simulink model "MCVT_Integration"  (R2020a)
settings: Solver=ode8  StartTime=0.0  StopTime=T_end  FixedStep=dt  RelTol=1e-3  EnableMultiTasking=on
values from neighbouring files (symbols this model uses):
  assumed_power_voltage = 1.25e2    (= 125)    [MCVT_Input_File.m]    (also in MCVT_Run_QuickCheck.m)
  battery_power_toggle = 1    [MCVT_Input_File.m]    (also in MCVT_Run_QuickCheck.m)
  battery_trickle_charge_param = 0.1    [MCVT_Input_File.m]    (also in MCVT_Run_QuickCheck.m)
  battery_trickle_leakage_param = 0.01    [MCVT_Input_File.m]    (also in MCVT_Run_QuickCheck.m)
  damage_case_id = 3    [MCVT_Input_File.m]    (also in MCVT_Run_QuickCheck.m = 1)
  dt = 1/fs    (= 0.001)    [MCVT_Run_QuickCheck.m]    (also in MCVT_Sim_Set.m)
  dt_2 = 1/fs_2    (= 0.001)    [MCVT_Run_QuickCheck.m]    (also in MCVT_Sim_Set.m)
  dt_3 = 1/fs_3    (= 0.001)    [MCVT_Run_QuickCheck.m]    (also in MCVT_Sim_Set.m)
  dt_5 = 1/fs_5    (= 0.005)    [MCVT_Run_QuickCheck.m]    (also in MCVT_Sim_Set.m)
  dt_8 = 1/fs_8    (= 0.05)    [MCVT_Run_QuickCheck.m]    (also in MCVT_Sim_Set.m)
  dt_9 = 1/fs_9    (= 0.001)    [MCVT_Run_QuickCheck.m]    (also in MCVT_Sim_Set.m)
  dust_met_duration = 3    [MCVT_Input_File.m]    (also in MCVT_Run_QuickCheck.m = 2)
  ECLSS_indoor_set_temperature = 298.15    [MCVT_Input_File.m]    (also in MCVT_Run_QuickCheck.m)
  energy_store_max_o = 150    [MCVT_Input_File.m]    (also in MCVT_Run_QuickCheck.m)
  energy_store_o = 120    [MCVT_Input_File.m]    (also in MCVT_Run_QuickCheck.m)
  fs = 1000    [MCVT_Run_QuickCheck.m]    (also in MCVT_Sim_Set.m)
  gain_charge_param = 1    [MCVT_Input_File.m]    (also in MCVT_Run_QuickCheck.m)
  ground_temperature = 373    [MCVT_Habitat_Design_Input_File.m]    (also in MCVT_Run_QuickCheck.m)
  I_max_charge = 800    [MCVT_Input_File.m]    (also in MCVT_Run_QuickCheck.m)
  I_max_discharge = 800    [MCVT_Input_File.m]    (also in MCVT_Run_QuickCheck.m)
  initial_pressure = 1e5    (= 100000)    [MCVT_Input_File.m]    (also in MCVT_Run_QuickCheck.m)
  initial_temperature = 295    [MCVT_Input_File.m]    (also in MCVT_Run_QuickCheck.m)
  interior_leak_rate = 0.00415    [MCVT_Input_File.m]    (also in MCVT_Run_QuickCheck.m)
  launch_dust_accum_start_time = 10    [MCVT_Input_File.m]    (also in MCVT_Run_QuickCheck.m = 2)
  launch_dust_accum_stop_time = 12    [MCVT_Input_File.m]    (also in MCVT_Run_QuickCheck.m = 3)
  meteorite_impact_time = 2    [MCVT_Input_File.m]    (also in MCVT_Run_QuickCheck.m = 1)
  nuclear_power_toggle = 1    [MCVT_Input_File.m]    (also in MCVT_Run_QuickCheck.m)
  print_interval = 30    [MCVT_Input_File.m]
  SOC_lower_thres = 0.5    [MCVT_Input_File.m]    (also in MCVT_Run_QuickCheck.m)
  SOC_upper_thres = 0.9    [MCVT_Input_File.m]    (also in MCVT_Run_QuickCheck.m)
  solar_power_toggle = 1    [MCVT_Input_File.m]    (also in MCVT_Run_QuickCheck.m)
  space_temperature = 4    [MCVT_Habitat_Design_Input_File.m]    (also in MCVT_Run_QuickCheck.m)
  Start_angle = 90    [MCVT_Input_File.m]    (also in MCVT_Run_QuickCheck.m)
undefined symbols (not defined in the file or in its folder): A_flow_restrictor, ACTIVITY_LOOKUP_TABLE, air, Aref, Bmap, Bmap2, Bmap3, comms, DiscIntNeverNeededParam, DmgSPL, dust_parameters, ECLSS_dust_damage_repair_time, ECLSS_heater_capacity, ECLSS_IPS_restrictor, ECLSS_IPS_setpointPressure, ECLSS_paint_damage_repair_time, ECLSS_radiatorLoop_discreteNumber, ECLSS_Vst, emptyActivity, expected_heat_add, FI, habitat, HIEM2SMM, HIEM2STM, HIEMDamageElements, impact_numbering, initial_SPLT_damage_index, input_air, input_Cond, input_CP, input_discretization, input_EV, input_Evap, input_radiatorLoop, K_ECLSS_dust_single, K_ECLSS_paint_single, K_Structure_damage_single, Kref, kW_to_W, meteoroidDustDep, mFRF, ndelays, nominalDustDep, noverlap, nuclear_toggle_gain, output_limits, panel_paint0, Ps0, reference_temperature, RepairTime_ECLSS_factor, Sensor_Location_SMM, SMM_T0, SMM_T1, SMM_T2, SMM_T3, SMM_T4, SMM_T4_0, SMM_T4_1, SMM_T4_2, solar_FDD_K ... (+73)
libraries: neural x350, simulink x39, xpcutilitieslib x7, xpcrtudplib x7, dspstat3 x1
(part 1 of 43)
settings Model: Creator=cjpan  LastModifiedBy=Awareness
settings SolverCC: ConcurrentTasks=on  AutoInsertRateTranBlk=on
settings DataIOCC: SaveFormat=StructureWithTime  ReturnWorkspaceOutputs=off
settings OptimizationCC: BlockReduction=off  OptimizeBlockIOStorage=off  BufferReuse=off  GlobalBufferReuse=off  ExpressionFolding=off  LocalBlockOutputs=off  OptimizeDataStoreBuffers=off  BusAssignmentInplaceUpdate=off
settings DebuggingCC: ArithmeticOperatorsInVariantConditions=warning
settings SFSimCC: CustomCodeUndefinedFunction=UseInterfaceOnly
settings RTWCC: Description=Generic Real-Time Target  RTWVerbose=off  BuildConfiguration=Faster Runs  RTWCompilerOptimization=on
settings GRTTargetCC: TargetLangStandard=C89/C90 (ANSI)

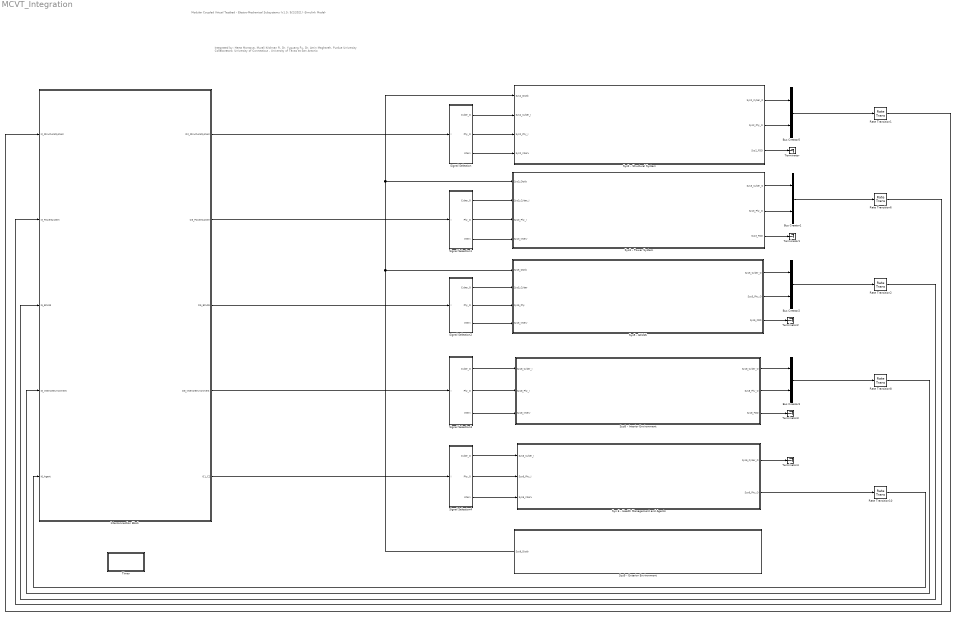
== system MCVT_Integration ==
  note: Modular Coupled Virtual Testbed - Electro-Mechanical Subsystems (v1.5: 9/2/2021)
      | -Simulink Model-
  note: Integrated by: Herta Montoya, Murali Krishnan R, Dr. Yuguang Fu, Dr. Amin Maghareh, Purdue University
      | Collaborators: University of Connecticut , University of Texas at San Antonio
  Bus Creator{1..3,5} (4 alike) : BusCreator  Inputs=2
  Interconnection Block : Subsystem  mask(Interconnection Block){}
  Rate Transition{1..2,6,8,10} (5 alike) : RateTransition  OutPortSampleTime=dt
  Signal Selection : Subsystem
  Signal Selection1 : Subsystem
  Signal Selection2 : Subsystem
  Signal Selection3 : Subsystem
  Signal Selection4 : Subsystem
  Sys 6 - Health Management and Agents : Subsystem  mask(Agents and C2){}
      mask help: Provide a summary of functionalities here
  Sys2 - Structural System : Subsystem  mask(Subsystem 2: Structure){}
      mask help:
      | - Structural Mechanical
      | - Structural Thermal
      | - Structural Protective Layer
      | - Structural Mechanical FDD
  Sys3 - Power System : Subsystem  mask(Subsystem 3: Power){}
      mask help: Provide a summary of functionalities here
  Sys5 - ECLSS : Subsystem  mask(Subsystem 5: ECLSS){}
      mask help: Provide a summary of functionalities here
  Sys8 - Interior Environment : Subsystem  mask(Subsystem 8: Interior Environment){}
      mask help: Provide a summary of functionalities here
  Sys9 - Exterior Environment : Subsystem  mask(Subsystem 9: Exterior Environment){}
      mask help: Provide a summary of functionalities here
  Terminator{,1..4} (5 alike) : Terminator
  Timer : Subsystem
  wires:
  Rate Transition2/1 -> Interconnection Block/3(I5_ECLSS)
  Rate Transition6/1 -> Interconnection Block/2(I3_PowerSystem)
  Rate Transition8/1 -> Interconnection Block/4(I8_InteriorEnvironment)
  Rate Transition10/1 -> Interconnection Block/5(I6_Agent)
  Sys9 - Exterior Environment/1(Sys9_Distb) -> Sys2 - Structural System/1(Sys2_Distb), Sys3 - Power System/1(Sys3_Distb), Sys5 - ECLSS/1(Sys5_Distb)
  Sys 6 - Health Management and Agents/2(Sys6_Phy_O) -> Rate Transition10/1
  Interconnection Block/2(O3_PowerSystem) -> Signal Selection1/1(I)
  Bus Creator5/1 -> Rate Transition1/1
  Sys2 - Structural System/2(Sys2_Phy_O) -> Bus Creator5/2
  Sys2 - Structural System/1(Sys2_Cyber_O) -> Bus Creator5/1
  Sys3 - Power System/1(Sys3_Cyber_O) -> Bus Creator1/1
  Sys3 - Power System/2(Sys3_Phy_O) -> Bus Creator1/2
  Bus Creator1/1 -> Rate Transition6/1
  Sys5 - ECLSS/2(Sys5_Phy_O) -> Bus Creator2/2
  Sys5 - ECLSS/1(Sys5_Cyber_O) -> Bus Creator2/1
  Bus Creator2/1 -> Rate Transition2/1
  Signal Selection/2(Phy_O) -> Sys2 - Structural System/3(Sys2_Phy_I)
  Signal Selection/3(Interv) -> Sys2 - Structural System/4(Sys2_Interv)
  Signal Selection/1(Cyber_O) -> Sys2 - Structural System/2(Sys2_Cyber_I)
  Signal Selection1/1(Cyber_O) -> Sys3 - Power System/2(Sys3_Cyber_I)
  Signal Selection1/2(Phy_O) -> Sys3 - Power System/3(Sys3_Phy_I)
  Signal Selection1/3(Interv) -> Sys3 - Power System/4(Sys3_Interv)
  Interconnection Block/3(O5_ECLSS) -> Signal Selection2/1(I)
  Signal Selection2/1(Cyber_O) -> Sys5 - ECLSS/2(Sys5_Cyber)
  Signal Selection2/2(Phy_O) -> Sys5 - ECLSS/3(Sys5_Phy)
  Interconnection Block/4(O8_InteriorEnvrionment) -> Signal Selection3/1(I)
  Signal Selection3/1(Cyber_O) -> Sys8 - Interior Environment/1(Sys8_Cyber_I)
  Signal Selection3/2(Phy_O) -> Sys8 - Interior Environment/2(Sys8_Phy_I)
  Signal Selection3/3(Interv) -> Sys8 - Interior Environment/3(Sys8_Interv)
  Interconnection Block/5(O1_C2) -> Signal Selection4/1(I)
  Sys8 - Interior Environment/1(Sys8_Cyber_O) -> Bus Creator3/1
  Sys8 - Interior Environment/2(Sys8_Phy_O) -> Bus Creator3/2
  Bus Creator3/1 -> Rate Transition8/1
  Signal Selection4/1(Cyber_O) -> Sys 6 - Health Management and Agents/1(Sys6_Cyber_I)
  Signal Selection4/2(Phy_O) -> Sys 6 - Health Management and Agents/2(Sys6_Phy_I)
  Signal Selection4/3(Interv) -> Sys 6 - Health Management and Agents/3(Sys6_Interv)
  Rate Transition1/1 -> Interconnection Block/1(I2_StructuralSystem)
  Interconnection Block/1(O2_StructuralSystem) -> Signal Selection/1(I)
  Sys2 - Structural System/3(Sys2_FDD) -> Terminator/1
  Sys3 - Power System/3(Sys3_FDD) -> Terminator1/1
  Sys5 - ECLSS/3(Sys5_FDD) -> Terminator2/1
  Sys8 - Interior Environment/3(Sys8_FDD) -> Terminator3/1
  Sys 6 - Health Management and Agents/1(Sys6_Cyber_O) -> Terminator4/1
  Signal Selection2/3(Interv) -> Sys5 - ECLSS/4(Sys5_Interv)

== system MCVT_Integration/Interconnection Block ==
  note: - System Interdependencies | Signal Exchange -
  note: Outputs from the Interconnection Block to the Structural System
  note: Interconnection Block
  note: Inputs from the Structural System to the Interconnection Block
  note: Inputs from the Power System to the Interconnection Block
  note: Outputs from the Interconnection Block to the Power System
  note: Inputs from ECLSS to the Interconnection Block
  note: Outputs from the Interconnection Block to ECLSS
  note: Inputs from the Interior Environment to the Interconnection Block
  note: Outputs from the Interconnection Block to the Interior Environment
  note: Outputs from the Interconnection Block to C2/Agent
  note: Inputs from the Agent to the Interconnection Block
  I2_StructuralSystem : Inport
  I3_PowerSystem : Inport  Port=2
  I5_ECLSS : Inport  Port=3
  I8_InteriorEnvironment : Inport  Port=4
  I6_Agent : Inport  Port=5
  Bus Creator : BusCreator  Inputs=4
  Bus Creator1 : BusCreator  Inputs=5
  Bus Creator2 : BusCreator  Inputs=10
  Bus Creator3 : BusCreator  Inputs=5
  Bus Selector : BusSelector  OutputSignals=Sys3_PhyO.Sys3_Out_Power_Supply_to_Sys2_kW_
  Bus Selector1 : BusSelector  OutputSignals=Sys8_PhyO.Sys8_Out_TempWall,Sys8_PhyO.Sys8_Out_PressureWall
  Bus Selector10 : BusSelector  OutputSignals=Sys8_CyberO.Sys8_Out_PSTemp,Sys8_PhyO.Sys8_Out_Airflow,Sys8_CyberO.Sys8_Out_PCPressure
  Bus Selector11 : BusSelector  OutputSignals=AgentComms.Repair_Comms_NPG,AgentComms.Repair_Comms_SPG
  Bus Selector12 : BusSelector  OutputSignals=Sys2_CyberO.Sys2_out_PowerConsumption
  Bus Selector2 : BusSelector  OutputSignals=Sys3_PhyO.Sys3_Out_Power_Supply_to_Sys5_kW_
  Bus Selector3 : BusSelector  OutputSignals=AgentComms.Repair_Comms_Structure
  Bus Selector4 : BusSelector  OutputSignals=Sys3_PhyO.Sys3_Out_Power_Supply_to_Sys8_kW_
  Bus Selector5 : BusSelector  OutputSignals=Sys5_PhyO.Sys5_Out_Airflow,Sys5_PhyO.Sys5_Out_Temp,Sys5_PhyO.Sys5_Air_Vent,Sys5_PhyO.Sys5_Air_Supply,Sys5_PhyO.Sys5_Out_HeatingEnergy,Sys5_PhyO.Sys5_Out_Heat
  Bus Selector6 : BusSelector  OutputSignals=AgentComms.Repair_Comms_ECLSS
  Bus Selector7 : BusSelector  OutputSignals=Sys2_PhyO.Sys2_out_InteriorWallSurfTemp,Sys2_PhyO.Sys2_out_Damage,Sys2_PhyO.Sys2_out_ExteriorWallSurfTemp
  Bus Selector8 : BusSelector  OutputSignals=Sys5_CyberO.Sys5_Out_PowerRequest
  Bus Selector9 : BusSelector  OutputSignals=Sys8_CyberO.Sys8_Out_PowerConsumption
  C2 Connection Handler : Subsystem
  O2_StructuralSystem : Outport
  O3_PowerSystem : Outport  Port=2
  O5_ECLSS : Outport  Port=3
  O8_InteriorEnvrionment : Outport  Port=4
  O1_C2 : Outport  Port=5
  wires:
  I3_PowerSystem/1 -> [Power]
  I8_InteriorEnvironment/1 -> [InteriorEnv]
  Bus Selector/1 -> Bus Creator/1  "<Sys3_Out_Power_Supply_to_Sys2_kW_>"
  Bus Selector1/1 -> Bus Creator/2  "<Sys8_Out_TempWall>"
  Bus Creator/1 -> O2_StructuralSystem/1
  Bus Selector2/1 -> Bus Creator1/1  "<Sys3_Out_Power_Supply_to_Sys5_kW_>"
  Bus Creator1/1 -> O5_ECLSS/1
  I5_ECLSS/1 -> [ECLSS]
  Bus Creator2/1 -> O8_InteriorEnvrionment/1
  I2_StructuralSystem/1 -> [Structural]
  Bus Selector4/1 -> Bus Creator2/4  "<Sys3_Out_Power_Supply_to_Sys8_kW_>"
  Bus Selector5/1 -> Bus Creator2/5  "<Sys5_Out_Airflow>"
  Bus Selector5/2 -> Bus Creator2/6  "<Sys5_Out_Temp>"
  Bus Selector8/1 -> Bus Creator3/2  "<Sys5_Out_PowerRequest>"
  Bus Selector9/1 -> Bus Creator3/3  "<Sys8_Out_PowerConsumption>"
  Bus Creator3/1 -> O3_PowerSystem/1
  Bus Selector5/3 -> Bus Creator2/7  "<Sys5_Air_Vent>"
  Bus Selector5/4 -> Bus Creator2/8  "<Sys5_Air_Supply>"
  Bus Selector10/1 -> Bus Creator1/2  "<Sys8_Out_PSTemp>"
  Bus Selector10/2 -> Bus Creator1/3  "<Sys8_Out_Airflow>"
  Bus Selector10/3 -> Bus Creator1/4  "<Sys8_Out_PCPressure>"
  Bus Selector5/5 -> Bus Creator2/9  "<Sys5_Out_HeatingEnergy>"
  Bus Selector1/2 -> Bus Creator/3  "<Sys8_Out_PressureWall>"
  I6_Agent/1 -> [Agent]
  Bus Selector3/1 -> Bus Creator/4  "<Repair_Comms_Structure>"
  Bus Selector11/1 -> Bus Creator3/4  "<Repair_Comms_NPG>"
  Bus Selector11/2 -> Bus Creator3/5  "<Repair_Comms_SPG>"
  C2 Connection Handler/1(O1_C2) -> O1_C2/1
  Bus Selector5/6 -> Bus Creator2/10  "<Sys5_Out_Heat>"
  [Structural] -> Bus Selector12/1
  Bus Selector12/1 -> Bus Creator3/1  "<Sys2_out_PowerConsumption>"
  [Structural] -> Bus Selector7/1
  Bus Selector7/1 -> Bus Creator2/1  "<Sys2_out_InteriorWallSurfTemp>"
  Bus Selector7/2 -> Bus Creator2/2  "<Sys2_out_Damage>"
  Bus Selector7/3 -> Bus Creator2/3  "<Sys2_out_ExteriorWallSurfTemp>"
  [Structural] -> C2 Connection Handler/1(I2_StructuralSystem)
  [Power] -> Bus Selector/1
  [Power] -> Bus Selector2/1
  [Power] -> Bus Selector4/1
  [Power] -> C2 Connection Handler/2(I2_PowerSystem)
  [ECLSS] -> Bus Selector8/1
  [ECLSS] -> Bus Selector5/1
  [ECLSS] -> C2 Connection Handler/3(I5_ECLSS)
  [InteriorEnv] -> Bus Selector1/1
  [InteriorEnv] -> Bus Selector9/1
  [InteriorEnv] -> Bus Selector10/1
  [Agent] -> Bus Selector3/1
  [Agent] -> Bus Selector11/1
  [Agent] -> Bus Selector6/1
  Bus Selector6/1 -> Bus Creator1/5  "<Repair_Comms_ECLSS>"

== system MCVT_Integration/Signal Selection ==
  I : Inport
  Bus Creator : BusCreator  Inputs=3
  Bus Selector : BusSelector  OutputSignals=Sys8_Out_TempWall,Sys8_Out_PressureWall,Sys3_Out_Power_Supply_to_Sys2_kW_
  Bus Selector1 : BusSelector  OutputSignals=Repair_Comms_Structure
  Cyber_O : Outport
  Phy_O : Outport  Port=2
  Interv : Outport  Port=3
  wires:
  Bus Selector1/1 -> Interv/1  "<Repair_Comms_Structure>"
  I/1 -> Bus Selector1/1, Bus Selector/1
  Bus Creator/1 -> Phy_O/1
  Bus Selector/1 -> Bus Creator/1  "<Sys8_Out_TempWall>"
  Bus Selector/2 -> Bus Creator/2  "<Sys8_Out_PressureWall>"
  Bus Selector/3 -> Bus Creator/3  "<Sys3_Out_Power_Supply_to_Sys2_kW_>"

== system MCVT_Integration/Signal Selection1 ==
  I : Inport
  Bus Creator : BusCreator  Inputs=3
  Bus Creator1 : BusCreator  Inputs=2
  Bus Selector : BusSelector  OutputSignals=Sys2_out_PowerConsumption,Sys5_Out_PowerRequest,Sys8_Out_PowerConsumption
  Bus Selector1 : BusSelector  OutputSignals=Repair_Comms_NPG,Repair_Comms_SPG
  Cyber_O : Outport
  Phy_O : Outport  Port=2
  Interv : Outport  Port=3
  wires:
  I/1 -> Bus Selector/1, Bus Selector1/1
  Bus Selector/1 -> Bus Creator/1  "<Sys2_out_PowerConsumption>"
  Bus Selector/2 -> Bus Creator/2  "<Sys5_Out_PowerRequest>"
  Bus Selector/3 -> Bus Creator/3  "<Sys8_Out_PowerConsumption>"
  Bus Creator/1 -> Cyber_O/1
  Bus Selector1/1 -> Bus Creator1/1  "<Repair_Comms_NPG>"
  Bus Selector1/2 -> Bus Creator1/2  "<Repair_Comms_SPG>"
  Bus Creator1/1 -> Interv/1

== system MCVT_Integration/Signal Selection2 ==
  I : Inport
  Bus Creator : BusCreator  Inputs=2
  Bus Creator1 : BusCreator  Inputs=2
  Bus Creator2 : BusCreator  Inputs=2
  Bus Selector : BusSelector  OutputSignals=Sys8_Out_PSTemp,Sys8_Out_PCPressure
  Bus Selector1 : BusSelector  OutputSignals=Sys3_Out_Power_Supply_to_Sys5_kW_,Sys8_Out_Airflow
  Bus Selector2 : BusSelector  OutputSignals=Repair_Comms_ECLSS
  Subsystem : Subsystem
  Cyber_O : Outport
  Phy_O : Outport  Port=2
  Interv : Outport  Port=3
  wires:
  I/1 -> Bus Selector1/1, Bus Selector/1, Bus Selector2/1
  Bus Selector/1 -> Bus Creator/1  "<Sys8_Out_PSTemp>"
  Bus Selector/2 -> Bus Creator/2  "<Sys8_Out_PCPressure>"
  Bus Creator/1 -> Cyber_O/1
  Bus Selector1/1 -> Bus Creator1/1  "<Sys3_Out_Power_Supply_to_Sys5_kW_>"
  Bus Selector1/2 -> Bus Creator1/2  "<Sys8_Out_Airflow>"
  Bus Creator1/1 -> Phy_O/1
  Bus Creator2/1 -> Interv/1  "Sys5_Interv"
  Bus Selector2/1 -> Subsystem/1(<Repair_Comms_ECLSS>)  "<Repair_Comms_ECLSS>"
  Subsystem/1(Repair_ECLSS_Dust) -> Bus Creator2/1
  Subsystem/2(Repair_ECLSS_Paint) -> Bus Creator2/2

== system MCVT_Integration/Signal Selection3 ==
  I : Inport
  Cyber_O : Outport
  Phy_O : Outport  Port=2
  Interv : Outport  Port=3
  wires:
  I/1 -> Phy_O/1

== system MCVT_Integration/Signal Selection4 ==
  I : Inport
  Bus Selector : BusSelector  OutputSignals=Sys6_Cyber,Sys6_Physical
  Cyber_O : Outport
  Phy_O : Outport  Port=2
  Interv : Outport  Port=3
  wires:
  I/1 -> Bus Selector/1
  Bus Selector/1 -> Cyber_O/1  "<Sys6_Cyber>"
  Bus Selector/2 -> Phy_O/1  "<Sys6_Physical>"

== system MCVT_Integration/Sys 6 - Health Management and Agents ==
  note: Coded by: Murali Krishnan Rajasekharan Pillai
      | Affiliations: Purdue University
      | Funded by: NASA STRI - RETH Institute
      |
      | Current Features (for the Agent Model):
      | - Single Agent Model with Finite Memory and FIFO Scheduling with no over-ride
      | - Agent States : [soc, position, mobility-HS]
      | - Agent Interactions : [Nuclear PG, Solar PG, Structure, ECLSS, Inventory]
      |
      | Current featurers (for the Decision Maker)
      | - FIFO scheduler which cann potentially re-schedule activities if FDD component doesn't react fast to the intervention
      | - No over-ride functionality covered (aka, cannot `cancel` an intervention), except at Habitat design level (aka, within the `MCVT_Habitat_Input_File`)
  note: Health Management System and Agent Model
  note: Systems Outputs to File
  Sys6_Cyber_I : Inport
  Sys6_Phy_I : Inport  Port=2
  Sys6_Interv : Inport  Port=3
  " Agent System" : Subsystem
  Bus Creator : BusCreator  Inputs=1
  Bus Creator1 : BusCreator  Inputs=1
  Bus Creator2 : BusCreator  Inputs=2
  Decision Maker : Subsystem
      settings: PortSchema="{\"entries\":[{\"content\":{\"sides\":[{\"content\":{\"connectorIds\":[\"In1\"]},\"type\":\"ConnectorPlacement.EquallySpacedRectSide\",\"uuid\":\"af73862b-44c1-41ec-b3ca-270812b76194\"},{\"content\":{\"connectorIds\":[\"In2\",\"In3\"],\"side\":\"RIGHT\"},\"type\":\"ConnectorPlacement.EquallySpacedRectSide\",\"uuid\":\"9454815f-a3fd-4c8a-9f4b-2b34d3ab7b42\"},{\"content\":{\"connectorIds\":[],\"side\":\"TOP\"},\"type\":\"ConnectorPlacement.EquallySpacedRectSide\",\"uuid\":\"90a25189-81d9-48d5-b6fa-d5f2bdc0713f\"},{\"content\":{\"connectorIds\":[\"Out1\"],\"side\":\"BOTTOM\"},\"type\":\"ConnectorPlacement.EquallySpacedRectSide\",\"uuid\":\"1751f83f-c6df-4ec8-9c75-9827cc634605\"}]},\"type\":\"ConnectorPlacement.EquallySpacedConnector\",\"uuid\":\"55de7abc-ed98-425f-94f2-679ed52f4cec\"}],\"packageUris\":[\"http://schema.mathworks.com/mf0/Connector/19700101\"],\"version\":\"1.0\"}"
  Rate Transition1 : RateTransition  OutPortSampleTime=dt_6
  Rate Transition9 : RateTransition  OutPortSampleTime=dt_6
  Terminator2 : Terminator
  To File : ToFile  Filename=Sys6_out.mat  MatrixName=sys6_out  SaveFormat=Timeseries
  Sys6_Cyber_O : Outport
  Sys6_Phy_O : Outport  Port=2
  wires:
  Rate Transition9/1 -> Decision Maker/3(Sub6_Cyber_Inputs)
  " Agent System"/1(AgentStates) -> Decision Maker/1(Agent_States), Bus Creator/1  "Agent_States"
  Sys6_Cyber_I/1 -> Rate Transition9/1
  Sys6_Interv/1 -> Terminator2/1
  Bus Creator/1 -> Sys6_Cyber_O/1, [Sys6_CyberO]  "Sys6_CyberO"
  Decision Maker/1(Intervention_Pgm) -> " Agent System"/1(Intervention_Pgm)
  Sys6_Phy_I/1 -> Rate Transition1/1
  Bus Creator1/1 -> [Sys6_PhysO], Sys6_Phy_O/1  "Sys6_Phy_O"
  " Agent System"/2(AgentComms) -> Bus Creator1/1  "AgentComms"
  Bus Creator2/1 -> To File/1
  [Sys6_CyberO] -> Bus Creator2/1  "Sys6_CyberO"
  [Sys6_PhysO] -> Bus Creator2/2  "Sys6_PhyO"
  Rate Transition1/1 -> Decision Maker/2(Sys6_Phy_Inputs)

== system MCVT_Integration/Sys2 - Structural System ==
  note: Coded by: Dr . Seungwook Seok, Adnan Shahriar, Sterling Reynolds, Sachin Tripathi, Jeffrey Steiner, Zixin Wang
      | Affiliations: University of Texas at San Antonio , University of Connecticut, Purdue University
  note: Structural System
      | - Simulink Model -
  note: Systems Outputs to File
  Sys2_Distb : Inport
  Sys2_Cyber_I : Inport  Port=2
  Sys2_Phy_I : Inport  Port=3
  Sys2_Interv : Inport  Port=4
  Bus Creator1 : BusCreator  Inputs=3
  Bus Creator2 : BusCreator  Inputs=2
  Bus Creator6 : BusCreator  Inputs=4
  Bus Selector : BusSelector  OutputSignals=SolarFluxSpatial,GroundAcc_xyz,MetCord_xyz,FI_index,dmg_caseo
  Bus Selector1 : BusSelector  OutputSignals=Sys8_Out_TempWall,Sys8_Out_PressureWall,Sys3_Out_Power_Supply_to_Sys2_kW_
  Rate Transition1 : RateTransition  OutPortSampleTime=dt_2
  Rate Transition2 : RateTransition  OutPortSampleTime=dt_2
  Rate Transition3 : RateTransition  OutPortSampleTime=dt_2
  Sys2_Structural_System : Subsystem
  Terminator : Terminator
  To File : ToFile  Filename=Sys2_out.mat  MatrixName=sys2_out  SaveFormat=Timeseries
  power2 : MATLAB Function  TreatAsAtomicUnit=on
  Sys2_Cyber_O : Outport
  Sys2_Phy_O : Outport  Port=2
  Sys2_FDD : Outport  Port=3
  wires:
  Sys2_Structural_System/7(Sys2_out_Total_Damage [-]) -> Bus Creator6/4  "Sys2_Total_Damage_to_Sys6"
  Rate Transition3/1 -> Sys2_Structural_System/9(Sys2_In_Repair_Status [-])
  Rate Transition1/1 -> Bus Selector/1
  Bus Creator1/1 -> [Sys2_CyberO], Sys2_Cyber_O/1  "Sys2_CyberO"
  Bus Selector/4 -> Sys2_Structural_System/4(Sys2_In_FI_Index [-])  "<FI_index>"
  Bus Selector1/2 -> Sys2_Structural_System/7(Sys2_In_Internal_Pressure [Pa])  "<Sys8_Out_PressureWall>"
  Rate Transition2/1 -> Bus Selector1/1
  Bus Selector/3 -> Sys2_Structural_System/3(Sys2_In_Meteoroid_Location [m])  "<MetCord_xyz>"
  Bus Selector1/1 -> Sys2_Structural_System/6(Sys2_In_Interior_Surface_Temperature [K])  "<Sys8_Out_TempWall>"
  Sys2_Structural_System/4(Sys2_out_InteriorWallSurfaceTemp [K]) -> Bus Creator6/1  "Sys2_out_InteriorWallSurfTemp"
  Sys2_Structural_System/5(Sys2_out_Damage [-]) -> Bus Creator6/2  "Sys2_out_Damage"
  Sys2_Distb/1 -> Rate Transition1/1
  Bus Selector/1 -> Sys2_Structural_System/1(Sys2_In_Solar_Irradiation [W/m^2])  "<SolarFluxSpatial>"
  Bus Selector/5 -> Sys2_Structural_System/5(Sys2_In_dmg_case [-])  "<dmg_caseo>"
  Sys2_Structural_System/2(Sys2_Out_Damage_Information [-]) -> [Sys2_FDD_Info], Bus Creator1/2  "Sys2_out_DamageProbabilities"
  power2/1(Y) -> Bus Creator1/3  "Sys2_out_PowerConsumption"
  Sys2_Phy_I/1 -> Rate Transition2/1
  Sys2_Structural_System/6(Sys2_out_ExteriorWallTemp [K]) -> Bus Creator6/3  "Sys2_out_ExteriorWallSurfTemp"
  Sys2_Structural_System/1(Sys2_out_InteriorWallSurfaceTemp_Sensors [K]) -> Bus Creator1/1  "Sys2_out_InteriorWallSurfTemp_ Sensors"
  Sys2_Structural_System/3(Sys2_out_PowerConsumption [kW]) -> power2/1(x)
  Sys2_Cyber_I/1 -> Terminator/1
  Sys2_Interv/1 -> Rate Transition3/1
  Bus Creator6/1 -> [Sys2_PhysO], Sys2_Phy_O/1  "Sys2_PhyO"
  Bus Selector1/3 -> Sys2_Structural_System/8(Sys2_In_Power_Supply [kW])  "<Sys3_Out_Power_Supply_to_Sys2_kW_>"
  Bus Selector/2 -> Sys2_Structural_System/2(Sys2_In_Moonquake_Acceleration [m/s^2])  "<GroundAcc_xyz>"
  Bus Creator2/1 -> To File/1
  [Sys2_FDD_Info] -> Sys2_FDD/1  "Sys2_FDD"
  [Sys2_PhysO] -> Bus Creator2/2  "Sys2_PhyO"
  [Sys2_CyberO] -> Bus Creator2/1  "Sys2_CyberO"

== system MCVT_Integration/Sys3 - Power System ==
  note: Systems Outputs to File
  note: Coded by: Ryan Tomastik, Kairui Hao
      | Affiliations: University of Connecticut, Purdue University
  note: Power System
      | -Simulink Model-
  Sys3_Distb : Inport
  Sys3_Cyber_I : Inport  Port=2
  Sys3_Phy_I : Inport  Port=3
  Sys3_Interv : Inport  Port=4
  Bus Creator2 : BusCreator  Inputs=2
  Bus Creator3 : BusCreator  Inputs=5
  Bus Creator4 : BusCreator  Inputs=2
  Bus Selector1 : BusSelector  OutputSignals=DustAccu,SolarFlux
  Bus Selector6 : BusSelector  OutputSignals=Sys2_out_PowerConsumption,Sys5_Out_PowerRequest,Sys8_Out_PowerConsumption
  Demux : Demux  Outputs=3
  Discrete-Time Integrator : DiscreteIntegrator  SampleTime=-1  ICPrevOutput=DiscIntNeverNeededParam  ICPrevScaledInput=DiscIntNeverNeededParam
  Power Generation Subsystem1 : Subsystem
  Rate Transition1 : RateTransition  OutPortSampleTime=dt_3
  Rate Transition4 : RateTransition  OutPortSampleTime=dt_3
  Rate Transition5 : RateTransition  OutPortSampleTime=dt_3
  Terminator : Terminator
  Terminator3 : Terminator
  To File : ToFile  Filename=Sys3_out.mat  MatrixName=sys3_out  SaveFormat=Timeseries
  Sys3_Cyber_O : Outport
  Sys3_Phy_O : Outport  Port=2
  Sys3_FDD : Outport  Port=3
  wires:
  Power Generation Subsystem1/3(Sys3_Out_Power_Supply_to_Sys8 [kW]) -> Bus Creator3/3  "Sys3_Out_Power_Supply_to_Sys8_kW_"
  Power Generation Subsystem1/2(Sys3_Out_Power_Supply_to_Sys5 [kW]) -> Bus Creator3/2  "Sys3_Out_Power_Supply_to_Sys5_kW_"
  Sys3_Cyber_I/1 -> Rate Transition5/1
  Demux/3 -> Terminator/1
  Sys3_Phy_I/1 -> Terminator3/1
  Power Generation Subsystem1/7(Sys3_Out_Current_Power_Stored [kWh]) -> Bus Creator2/2  "Sys3_Out_PowerStorage"
  Bus Creator2/1 -> [Sys3_CyberO], Sys3_Cyber_O/1  "Sys3_CyberO"
  Bus Selector6/2 -> Power Generation Subsystem1/5(Sys3_In_Power_Consumed_by_Sys5 [kW])  "<Sys5_Out_PowerRequest>"
  Bus Selector1/2 -> Power Generation Subsystem1/3(Sys3_In_Solar_Flux [W/m^2])  "<SolarFlux>"
  Bus Selector6/3 -> Power Generation Subsystem1/6(Sys3_In_Power_Consumed_by_Sys8 [kW])  "<Sys8_Out_PowerConsumption>"
  Rate Transition1/1 -> Power Generation Subsystem1/7(Sys3_In_Intervention)
  Bus Selector1/1 -> Demux/1  "<DustAccu>"
  [Sys3_CyberO] -> Bus Creator4/1  "Sys3_CyberO"
  Bus Creator4/1 -> To File/1
  Demux/2 -> Power Generation Subsystem1/2(Sys3_In_Dust_Accumulation_PV [mg/cm^2])
  Bus Selector6/1 -> Power Generation Subsystem1/4(Sys3_In_Power_Consumed_by_Sys2 [kW])  "<Sys2_out_PowerConsumption>"
  Power Generation Subsystem1/4(Sys3_Out_total_power [kW]) -> Bus Creator3/4  "Sys3_Out_Total_Power_Generated_kW_"
  Rate Transition4/1 -> Bus Selector1/1
  Sys3_Interv/1 -> Rate Transition1/1
  Power Generation Subsystem1/6(Sys3_Out_Damage_Information) -> [Sys3_FDD_Info], Bus Creator2/1  "Sys3_Out_PowerSystemDamageInformation"
  Sys3_Distb/1 -> Rate Transition4/1
  Bus Creator3/1 -> Sys3_Phy_O/1, [Sys3_PhysO]  "Sys3_PhyO"
  Power Generation Subsystem1/1(Sys3_Out_Power_Supply_to_Sys2 [kW]) -> Bus Creator3/1  "Sys3_Out_Power_Supply_to_Sys2_kW_"
  Power Generation Subsystem1/5(Sys3_Out_Physical_Inputs_Agents) -> Bus Creator3/5  "Sys3_Out_Physical_Inputs_Agents"
  Demux/1 -> Discrete-Time Integrator/1
  [Sys3_PhysO] -> Bus Creator4/2  "Sys3_PhyO"
  Rate Transition5/1 -> Bus Selector6/1
  Discrete-Time Integrator/1 -> Power Generation Subsystem1/1(Sys3_In_Dust_Accumulation_Nuclear [mg/cm^2])
  [Sys3_FDD_Info] -> Sys3_FDD/1  "Sys3_FDD"

== system MCVT_Integration/Sys5 - ECLSS ==
  note: Environmental Control and Life Support System
      | - Simulink Model -
  note: Coded by: Jaewon Park, Dr. C.J. Pan, Drake Hart
      | Affiliations: P urdue University
  note: %%%%todo%%%%
  note: Systems Outputs to File
  Sys5_Distb : Inport
  Sys5_Cyber : Inport  Port=2
  Sys5_Phy : Inport  Port=3
  Sys5_Interv : Inport  Port=4
  Active Thermal Control System (ATCS) : Subsystem
  Add : Sum
  Allocate Power : Sum  Inputs=+-
  Bus Creator1 : BusCreator  Inputs=2
  Bus Creator8 : BusCreator  Inputs=3
  Bus Creator9 : BusCreator  Inputs=6
  Bus Selector1 : BusSelector  OutputSignals=Sys8_Out_PSTemp,Sys8_Out_PCPressure
  Bus Selector2 : BusSelector  OutputSignals=SolarFlux,DustAccu,dmg_caseo
  Bus Selector3 : BusSelector  OutputSignals=Sys8_Out_Airflow,Sys3_Out_Power_Supply_to_Sys5_kW_
  Constant : Constant  Value=dt_5
  Demux1 : Demux  Outputs=3
  From Workspace : FromWorkspace  VariableName=[time_human activity_column]  SampleTime=dt_5
  From Workspace1 : FromWorkspace  VariableName=[time_human Location_id]  SampleTime=dt_5
  Human/System 5 : Subsystem
  IPCS_ setpoint pressure : Constant  Value=ECLSS_IPS_setpointPressure
  Interior Pressure Control System (IPCS) : Subsystem
  Mux : Mux  Inputs=2
  Mux3 : Mux  Inputs=3
  Rate Transition : RateTransition  OutPortSampleTime=dt_5
  Rate Transition1 : RateTransition  OutPortSampleTime=dt_5
  Rate Transition2 : RateTransition  InitialCondition=struct('Sys8_Out_PSTemp', initial_temperature-273.15, 'Sys8_Out_PCPressure',initial_pressure)  OutPortSampleTime=dt_5
  Rate Transition3 : RateTransition  OutPortSampleTime=dt_5
  Sys5.1_no_faulty_valve : Constant  Value=Valve_opening_factor
  Sys5_in_SetTemperature (C) : Constant  Value=Tset0
  Terminator{,1..3} (4 alike) : Terminator
  To File : ToFile  Filename=Sys5_out.mat  MatrixName=sys5_out  SaveFormat=Timeseries
  Sys5_Cyber_O : Outport
  Sys5_Phy_O : Outport  Port=2
  Sys5_FDD : Outport  Port=3
  wires:
  Sys5_Distb/1 -> Rate Transition/1
  Active Thermal Control System (ATCS)/9("Sys5_out_dust Health State (0: clean, 1: full damage)") -> Mux/2
  Bus Creator9/1 -> [Sys5_PhysO], Sys5_Phy_O/1  "Sys5_PhyO"
  From Workspace/1 -> Human/System 5/2(activity_id)
  From Workspace1/1 -> Human/System 5/3(Location_id)
  Active Thermal Control System (ATCS)/6(Sys5_out_airflow [m^3/s]) -> Bus Creator9/2  "Sys5_Out_Airflow"
  Allocate Power/1 -> Active Thermal Control System (ATCS)/6(Sys5_in_limited power supply [kW])
  Interior Pressure Control System (IPCS)/1(Sys5_out_air_released [kg/s]) -> Bus Creator9/4  "Sys5_Air_Vent"
  Bus Selector2/2 -> Demux1/1  "<DustAccu>"
  Active Thermal Control System (ATCS)/7(Sys5_out_evaporator temperature [C]) -> Bus Creator9/3  "Sys5_Out_Temp"
  Demux1/2 -> Terminator3/1
  Sys5.1_no_faulty_valve/1 -> Interior Pressure Control System (IPCS)/3(Sys5_in_faulty_valve [-])
  Bus Selector1/1 -> Active Thermal Control System (ATCS)/4(Sys5_in_indoor temperature [C])  "<Sys8_Out_PSTemp>"
  Bus Selector1/2 -> Interior Pressure Control System (IPCS)/2(Sys 5_in_interior pressure [Pa])  "<Sys8_Out_PCPressure>"
  Constant/1 -> Human/System 5/1(dt)
  Demux1/3 -> Active Thermal Control System (ATCS)/2(Sys5_in_dust_Input [g/cm^2/s])
  Active Thermal Control System (ATCS)/5(Sys5_out_heating_energy [kW]) -> Bus Creator9/1  "Sys5_Out_HeatingEnergy"
  Rate Transition2/1 -> Bus Selector1/1
  Sys5_Cyber/1 -> Rate Transition2/1
  Bus Selector2/3 -> Active Thermal Control System (ATCS)/3(Sys5_in_impact (1-4))  "<dmg_caseo>"
  Add/1 -> Bus Creator8/1  "Sys5_Out_PowerRequest"
  Active Thermal Control System (ATCS)/3(Sys5_out_power_request [kW]) -> Add/1
  Sys5_Interv/1 -> Rate Transition3/1
  Rate Transition1/1 -> Bus Selector3/1
  Sys5_in_SetTemperature (C)/1 -> Active Thermal Control System (ATCS)/7(Sys5_in_Tset [C])
  Rate Transition/1 -> Bus Selector2/1
  Active Thermal Control System (ATCS)/2(Sys5_out_sensor_data_Trs [C]) -> Terminator1/1
  Active Thermal Control System (ATCS)/4(Sys5_out_power consumption [kW]) -> Bus Creator8/2  "Sys5_ Out_ PowerConsumption"
  Interior Pressure Control System (IPCS)/3(Sys5_out_consumed_power [kW]) -> Allocate Power/2, Add/2
  Bus Creator8/1 -> [Sys5_CyberO], Sys5_Cyber_O/1  "Sys5_CyberO"
  Bus Selector3/1 -> Active Thermal Control System (ATCS)/5(Sys5_in_airflow through the evaporator [m^3/s])  "<Sys8_Out_Airflow>"
  Human/System 5/2(r) -> Mux3/2
  Active Thermal Control System (ATCS)/8("Sys5_out_paint Health State (0: clean, 1: full damage)") -> Mux/1
  Human/System 5/3(theta) -> Mux3/3
  Bus Selector3/2 -> Allocate Power/1  "<Sys3_Out_Power_Supply_to_Sys5_kW_>"
  Human/System 5/1(heat_profile_final) -> Mux3/1
  Interior Pressure Control System (IPCS)/2(Sys5_out_air_supply [kg/s]) -> Bus Creator9/5  "Sys5_Air_Supply"
  Active Thermal Control System (ATCS)/1(Sys5_out_sensor_data_Tfs [C]) -> Terminator/1
  IPCS_ setpoint pressure/1 -> Interior Pressure Control System (IPCS)/1(Sys5_in_setpoint_pressure [Pa])
  Sys5_Phy/1 -> Rate Transition1/1
  Demux1/1 -> Terminator2/1
  Bus Selector2/1 -> Active Thermal Control System (ATCS)/1(Sys5_in_solar radiation [W/m^2])  "<SolarFlux>"
  Mux3/1 -> Bus Creator9/6  "Sys5_Out_Heat"
  Mux/1 -> [Sys5_FDD_Info]
  [Sys5_FDD_Info] -> Sys5_FDD/1  "Sys5_FDD"
  Bus Creator1/1 -> To File/1
  [Sys5_PhysO] -> Bus Creator1/2  "Sys5_PhyO"
  [Sys5_CyberO] -> Bus Creator1/1  "Sys5_CyberO"
  Rate Transition3/1 -> Active Thermal Control System (ATCS)/10(Sys5_Repair_Status)
  Active Thermal Control System (ATCS)/10(Sys5_Out_Damage_Information) -> [Sys5_Out_Damage_Info]
  [Sys5_Out_Damage_Info] -> Bus Creator8/3  "Sys5_Out_Damage_Info"

== system MCVT_Integration/Sys8 - Interior Environment ==
  note: Interior Environment
      | -Simulink Model-
  note: Coded by: Jeffrey Steiner
      | Affiliations: University of Connecticut
  note: Systems Outputs to File
  Sys8_Cyber_I : Inport
  Sys8_Phy_I : Inport  Port=2
  Sys8_Interv : Inport  Port=3
  Bus Creator1 : BusCreator  Inputs=2
  Bus Creator11 : BusCreator  Inputs=3
  Bus Creator12 : BusCreator  Inputs=5
  Bus Selector5 : BusSelector  OutputSignals=Sys2_out_InteriorWallSurfTemp,Sys5_Out_Temp,Sys5_Out_Airflow,Sys5_Out_HeatingEnergy,Sys5_Air_Supply,Sys5_Air_Vent,Sys3_Out_Power_Supply_to_Sys8_kW_,Sys5_Out_Heat,Sys2_out_Damage
  Element : Constant  Value=40
  Element3 : Constant  Value=45
  Gain : Gain  Gain=-1
  Gain4 : Gain
  Gain6 : Gain  Gain=1000
  HIEM_Damage_Indication : MATLAB Function  TreatAsAtomicUnit=on
  Habitat Interior Environment Model : Subsystem
  Memory1 : Memory  InitialCondition=initial_temperature-273.15  InheritSampleTime=on
  Memory2 : Memory  InitialCondition=ones(60,1)*initial_temperature  InheritSampleTime=on
  Memory3 : Memory  InitialCondition=0.3  InheritSampleTime=on
  Memory4 : Memory  InitialCondition=initial_pressure  InheritSampleTime=on
  Memory5 : Memory  InitialCondition=ones(17,1)*initial_pressure  InheritSampleTime=on
  Memory6 : Memory  InitialCondition=ones(112,1)*initial_pressure  InheritSampleTime=on
  Memory7 : Memory  InitialCondition=ones(112,1)*initial_temperature  InheritSampleTime=on
  Rate Transition7 : RateTransition  OutPortSampleTime=dt_8
  Terminator{,1,3,5..6} (5 alike) : Terminator
  To File : ToFile  Filename=Sys8_out.mat  MatrixName=sys8_out  SaveFormat=Timeseries
  power1 : MATLAB Function  TreatAsAtomicUnit=on
  Sys8_Cyber_O : Outport
  Sys8_Phy_O : Outport  Port=2
  Sys8_FDD : Outport  Port=3
  wires:
  power1/1(Y) -> Bus Creator11/3  "Sys8_Out_PowerConsumption"
  Gain6/1 -> Habitat Interior Environment Model/4(Sys8_In_Heating [W])
  Bus Selector5/8 -> Habitat Interior Environment Model/8(Sys8_In_Humans)  "<Sys5_Out_Heat>"
  Sys8_Interv/1 -> Terminator6/1
  Element3/1 -> Habitat Interior Environment Model/11(Sys8_In_HeatingLoc [-])
  Sys8_Phy_I/1 -> Rate Transition7/1
  Habitat Interior Environment Model/5(Sys8_Out_AirPressure_ToIPCS [Pa]) -> Memory4/1
  Sys8_Cyber_I/1 -> Terminator5/1
  Memory4/1 -> Bus Creator11/2  "Sys8_Out_PCPressure"
  Habitat Interior Environment Model/4(Sys8_Out_AirTempECLSSWallSensor [K]) -> Memory1/1
  Bus Selector5/4 -> Gain6/1  "<Sys5_Out_HeatingEnergy>"
  Bus Creator12/1 -> [Sys8_PhysO], Sys8_Phy_O/1  "Sys8_PhyO"
  Gain/1 -> Habitat Interior Environment Model/6(Sys8_In_IPCS_Discharge [m^3/s])
  Habitat Interior Environment Model/9(Sys8_Out_AirPressure_ToSMM [Pa]) -> Memory5/1
  Bus Selector5/1 -> Habitat Interior Environment Model/1(Sys8_In_WallTemp [K])  "<Sys2_out_InteriorWallSurfTemp>"
  Memory5/1 -> Bus Creator12/3  "Sys8_Out_PressureWall"
  Habitat Interior Environment Model/2(Sys8_Out_IPCSAirflow [m^3/s]) -> Terminator1/1
  Element/1 -> Habitat Interior Environment Model/10(Sys8_In_CoolingLoc [-])
  Bus Selector5/5 -> Gain4/1  "<Sys5_Air_Supply>"
  Memory1/1 -> Bus Creator11/1  "Sys8_Out_PSTemp"
  Bus Selector5/2 -> Habitat Interior Environment Model/2(Sys8_In_Cooling [C])  "<Sys5_Out_Temp>"
  Bus Selector5/3 -> Habitat Interior Environment Model/3(Sys8_In_H/CAirFlow [m^3/s])  "<Sys5_Out_Airflow>"
  Bus Selector5/9 -> HIEM_Damage_Indication/1(DamageInfo)  "<Sys2_out_Damage>"
  Bus Selector5/7 -> Habitat Interior Environment Model/7(Sys8_In_SensorPowerSupply [kW])  "<Sys3_Out_Power_Supply_to_Sys8_kW_>"
  Habitat Interior Environment Model/1(Sys8_Out_AirTempWallSensor [K]) -> Terminator/1
  Gain4/1 -> Habitat Interior Environment Model/5(Sys8_In_IPCS_Supply [kg/s])
  Memory2/1 -> Bus Creator12/2  "Sys8_Out_TempWall"
  Bus Selector5/6 -> Gain/1  "<Sys5_Air_Vent>"
  Bus Creator11/1 -> [Sys8_CyberO], Sys8_Cyber_O/1  "Sys8_CyberO"
  Rate Transition7/1 -> Bus Selector5/1
  Habitat Interior Environment Model/8(Sys8_Out_AirTempWallToSTM [K]) -> Memory2/1
  Memory3/1 -> Bus Creator12/1  "Sys8_Out_Airflow"
  Bus Creator1/1 -> To File/1
  [Sys8_CyberO] -> Bus Creator1/1  "Sys8_CyberO"
  [Sys8_PhysO] -> Bus Creator1/2  "Sys8_PhyO"
  HIEM_Damage_Indication/1(Damage) -> Habitat Interior Environment Model/9(Sys8_In_Damage [-])
  Habitat Interior Environment Model/3(Sys8_Out_PSTemp [C]) -> Terminator3/1
  Habitat Interior Environment Model/6(Sys8_Out_SensorPowerConsumption [kW]) -> power1/1(x)
  Habitat Interior Environment Model/7(Sys8_Out_TMSAirflow [m^3/s]) -> Memory3/1
  Habitat Interior Environment Model/10(Sys8_Out_TotalAirPressureDistribution (Plotting and Testing)) -> Memory6/1
  Memory6/1 -> Bus Creator12/4  "Sys8_Out_TotalAirPressureDistribution"
  Habitat Interior Environment Model/11(Sys8_Out_AirTemp [K]) -> Memory7/1
  Memory7/1 -> Bus Creator12/5  "Sys8_Out_TotalAirTemperatureDistribution"

== system MCVT_Integration/Sys9 - Exterior Environment ==
  note: Coded by: Dr . Seungwook Seok, Sachin Tripathi, Dr. C.J. Pan, Dr. Amin Maghareh
      | Affiliations: University of Texas at San Antonio , University of Connecticut, Purdue University
  note: Exterior Environment
      | -Simulink Model-
  note: Systems Outputs to File
  Bus Creator4 : BusCreator  Inputs=7
  Clock1 : Clock
  Constant1 : Constant  Value=Sys9_int_EqMag  SampleTime=dt
  Exterior Environment : Subsystem  TreatAsAtomicUnit=on  SystemSampleTime=dt_9
  Gain2 : Gain  Gain=0
  Inclination Angle1 : Constant  Value=Sys9_int_SPAngle  SampleTime=dt
  Mux : Mux  Inputs=3
  Mux1 : Mux  Inputs=3
  Rate Transition3 : RateTransition  OutPortSampleTime=dt
  To File : ToFile  Filename=Sys9_out.mat  MatrixName=sys9_out  SaveFormat=Timeseries
  Sys9_Distb : Outport
  wires:
  Exterior Environment/2(Sys9_out_MetCordY) -> Mux/2
  Exterior Environment/10(Sys9_out_GroundAccY) -> Mux1/2
  Constant1/1 -> Exterior Environment/3(Sys9_in_Magnitude)
  Clock1/1 -> Exterior Environment/1(Sys9_in_Time)
  Exterior Environment/1(Sys9_out_MetCordX) -> Mux/1
  Exterior Environment/5(Sys9_out_dmg_case) -> Bus Creator4/3
  Mux/1 -> Bus Creator4/1  "MetCord_xyz"
  Exterior Environment/3(Sys9_out_MetCordZ) -> Mux/3
  Exterior Environment/4(Sys9_out_FI_index) -> Bus Creator4/2
  Bus Creator4/1 -> To File/1, Rate Transition3/1
  Mux1/1 -> Gain2/1
  Gain2/1 -> Bus Creator4/7  "GroundAcc_xyz"
  Exterior Environment/9(Sys9_out_GroundAccX) -> Mux1/1
  Exterior Environment/7(Sys9_out_SolarFlux) -> Bus Creator4/5  "SolarFlux"
  Exterior Environment/11(Sys9_out_GroundAccZ) -> Mux1/3
  Inclination Angle1/1 -> Exterior Environment/2(Sys9_in_InclinationAngle)
  Exterior Environment/8(Sys9_out_SolarFluxSpatial) -> Bus Creator4/6  "SolarFluxSpatial"
  Exterior Environment/6(Sys9_out_DustAccumulationLunch) -> Bus Creator4/4  "DustAccu"
  Rate Transition3/1 -> Sys9_Distb/1

== system MCVT_Integration/Timer ==
  Clock : Clock
  Constant : Constant  Value=print_interval
  MATLAB Function : MATLAB Function  TreatAsAtomicUnit=on
  wires:
  Clock/1 -> MATLAB Function/1(clock)
  Constant/1 -> MATLAB Function/2(print_interval)

== system MCVT_Integration/Interconnection Block/C2 Connection Handler ==
  note: States Unobserved by the HMS & Agents
  note: Real / Simulated FDDs of Electro-Mechanical Systems
  I2_StructuralSystem : Inport
  I2_PowerSystem : Inport  Port=2
  I5_ECLSS : Inport  Port=3
  Bus Creator : BusCreator  Inputs=2
  Bus Creator1 : BusCreator  Inputs=2
  Bus Creator4 : BusCreator  Inputs=3
  Bus Selector : BusSelector  OutputSignals=Sys2_PhyO.Sys2_Total_Damage_to_Sys6
  Bus Selector1 : BusSelector  OutputSignals=Sys3_PhyO.Sys3_Out_Physical_Inputs_Agents
  Bus Selector12 : BusSelector  OutputSignals=Sys2_CyberO.Sys2_out_DamageProbabilities
  Bus Selector13 : BusSelector  OutputSignals=Sys3_CyberO.Sys3_Out_PowerSystemDamageInformation
  Bus Selector2 : BusSelector  OutputSignals=Sys5_CyberO.Sys5_Out_Damage_Info
  O1_C2 : Outport
  wires:
  Bus Selector12/1 -> Bus Creator4/1  "<Sys2_out_DamageProbabilities>"
  I2_PowerSystem/1 -> [Power]
  Bus Selector13/1 -> Bus Creator4/2  "<Sys3_Out_PowerSystemDamageInformation>"
  I2_StructuralSystem/1 -> [Structural]
  Bus Creator4/1 -> Bus Creator1/1  "Sys6_Cyber"
  Bus Selector/1 -> Bus Creator/1  "<Sys2_Total_Damage_to_Sys6>"
  Bus Selector1/1 -> Bus Creator/2  "<Sys3_Out_Physical_Inputs_Agents>"
  Bus Creator/1 -> Bus Creator1/2  "Sys6_Physical"
  Bus Creator1/1 -> O1_C2/1
  [Structural] -> Bus Selector12/1
  [Power] -> Bus Selector13/1
  [Structural] -> Bus Selector/1
  [Power] -> Bus Selector1/1
  I5_ECLSS/1 -> [ECLSS]
  [ECLSS] -> Bus Selector2/1
  Bus Selector2/1 -> Bus Creator4/3  "<Sys5_Out_Damage_Info>"

== system MCVT_Integration/Signal Selection2/Subsystem ==
  <Repair_Comms_ECLSS> : Inport
  MATLAB Function : MATLAB Function  TreatAsAtomicUnit=on
  Scope : Scope  NumInputPorts=2
  Repair_ECLSS_Dust : Outport
  Repair_ECLSS_Paint : Outport  Port=2
  wires:
  MATLAB Function/1(Repair_ECLSS_Dust) -> Scope/1, Repair_ECLSS_Dust/1
  MATLAB Function/2(Repair_ECLSS_Paint) -> Repair_ECLSS_Paint/1, Scope/2
  <Repair_Comms_ECLSS>/1 -> MATLAB Function/1(Repair_Comms_ECLSS)

== system MCVT_Integration/Sys 6 - Health Management and Agents/ Agent System ==
  Intervention_Pgm : Inport
  Agent Module : Subsystem
  AgentStateBus : BusCreator  Inputs=3
  Interact_with_Susystems : Subsystem
  Viz : Subsystem
  AgentStates : Outport
  AgentComms : Outport  Port=2
  wires:
  Intervention_Pgm/1 -> Agent Module/1(Intervention_Pgm)
  AgentStateBus/1 -> AgentStates/1, Viz/1(AgentStates)  "AgentStates"
  Agent Module/2(Agent_Memory_States) -> AgentStateBus/2  "Agent_Memory_States"
  Agent Module/3(AgentPlantStates) -> AgentStateBus/3  "Agent_Plant_States"
  Agent Module/1(programInfo) -> AgentStateBus/1  "Agent_Program_Info"
  Agent Module/4(AgentComms) -> Interact_with_Susystems/1(Agent_SubSys_Comms)
  Interact_with_Susystems/1(Agent_Comms) -> AgentComms/1, Viz/2(Agent_Comms)

== system MCVT_Integration/Sys 6 - Health Management and Agents/Decision Maker ==
  note: EMS Module Integration Debug Module
  note: Reflexive Decision Maker
      | Description: Static, rule-based forward chaining expert system that processes
      | the FDD outputs of habitat systems to schedule intervention agents for repair
      | actions.
  Agent_States : Inport
  Sys6_Phy_Inputs : Inport  Port=2
  Sub6_Cyber_Inputs : Inport  Port=3
  Bus Selector1 : BusSelector  Commented=on  OutputSignals=Agent_Memory_States.Activity_IDs
  Clock : Clock
  Constant : Constant  Value=ACTIVITY_LOOKUP_TABLE
  Delay1 : Delay  DelayLength=1  InitialCondition=emptyActivity
  From : From  Commented=on  GotoTag=Agent_States
  From2 : From  Commented=on  GotoTag=Sub6_Cyber_Inputs
  MATLAB Function : MATLAB Function  TreatAsAtomicUnit=on
  Scope : Scope  Commented=on  NumInputPorts=2
  Simulink Communication Modules : Subsystem
  Viz : Subsystem
  module 2 : MATLABSystem  Commented=on  System=FifoDecisionMaker  acvtLookupTable=ACTIVITY_LOOKUP_TABLE  isAcvtActive=[1,1,1]  test_thresholds=[1, 1, 6]  test_modes=[3, 3, 7]  SimulateUsing=Code generation
      mask Type: FifoDecisionMaker
      mask Display:
      | disp('FifoDecisionMaker');
      | port_label('input',1,'acvtIds_inMemory');
      | port_label('input',2,'test_inputs');
      | port_label('output',1,'test_results');
      | port_label('output',2,'scheduled');
      | port_label('output',3,'suggested');
  module3 : Subsystem  Commented=on
  Intervention_Pgm : Outport
  wires:
  Agent_States/1 -> [Agent_States]
  Bus Selector1/1 -> module 2/1  "<Activity_IDs>"
  module3/1(test_inputs) -> module 2/2
  module 2/1 -> Scope/1
  module 2/2 -> Scope/2
  Constant/1 -> MATLAB Function/2(lookup_table)
  MATLAB Function/1(pgm) -> Delay1/1
  Clock/1 -> MATLAB Function/3(time)
  Sub6_Cyber_Inputs/1 -> [Sub6_Cyber_Inputs]
  Sys6_Phy_Inputs/1 -> [Sub6_Phy_Inputs]
  From/1 -> Bus Selector1/1
  [Sub6_Cyber_Inputs] -> Simulink Communication Modules/1(Sub6_Cyber_Inputs)
  From2/1 -> module3/1(Sub6_Cyber_Inputs)
  [Sub6_Cyber_Inputs] -> Viz/1(Sub6_Cyber_Inputs)
  [Sub6_Phy_Inputs] -> Viz/2(Sys6_Phy_Inputs)
  [Agent_States] -> Simulink Communication Modules/2(Agent_States)
  Delay1/1 -> Intervention_Pgm/1
  Simulink Communication Modules/1(HMS_Suggested_Activity) -> MATLAB Function/1(suggested_activity_id)
